simulink model "ofdm_txrx_mimo_2x"  (Simulink 6.6)
settings: Solver=VariableStepDiscrete  StartTime=0.0  StopTime=20e3  RelTol=1e-3
PreLoadFcn:
  | ofdm_rx_mimo_init
InitFcn:
  | ofdm_rx_mimo_init
StartFcn:
  | ofdm_rx_mimo_init
values from neighbouring files (symbols this model uses):
  BER_RAM_init_size = 2048    [ofdm_tx_mimo_init.m]
  CPLength = 16    [ofdm_tx_mimo_init.m]
  CRCPolynomial = hex2dec('04c11db7')    [ofdm_tx_mimo_init.m]
  freqOffset_track_filtCoef_i = hex2dec('2500')/2^32    [ofdm_rx_mimo_init.m]
  freqOffset_track_filtCoef_p = hex2dec('d0000')/2^32    [ofdm_rx_mimo_init.m]
  hi = zeros(1,length(cci))    [ofdm_rx_mimo_init.m]
  hr = zeros(1,length(ccr))    [ofdm_rx_mimo_init.m]
  max_CP_length = 16    [ofdm_tx_mimo_init.m]
  max_num_baseRateSymbols = 31    [ofdm_tx_mimo_init.m]
  max_num_subcarriers = 64    [ofdm_tx_mimo_init.m]
  max_num_trainingSymbols = 15    [ofdm_tx_mimo_init.m]
  max_numBytes = 16384    [ofdm_tx_mimo_init.m]
  max_OFDM_symbols = 2047    [ofdm_tx_mimo_init.m]
  modConstellation_qam16 = .75*[1 3 -1 -3]./3    [ofdm_tx_mimo_init.m]
  modConstellation_qam256 = 0.9375*[3 1 5 7 11 9 13 15 -3 -1 -5 -7 -11 -9 -13 -15]./15    [ofdm_tx_mimo_init.m]
  modConstellation_qam64 = 0.875*[3 1 5 7 -3 -1 -5 -7]./7    [ofdm_tx_mimo_init.m]
  modConstellation_qpsk = [1 -1]./sqrt(2)    [ofdm_tx_mimo_init.m]
  numBaseRateSymbols = 2    [ofdm_tx_mimo_init.m]
  numSubcarriers = 64    [ofdm_tx_mimo_init.m]
  numTrainingSymbols = 2    [ofdm_tx_mimo_init.m]
  pilot1_indicies = 7 + ( (64-7) * 2^16)    (= 3735559)    [ofdm_tx_mimo_init.m]
  pilot2_indicies = 21 + ( (64-21) * 2^16)    (= 2818069)    [ofdm_tx_mimo_init.m]
  pilotValue_neg = hex2dec('8000') + (2^16 * 0)    [ofdm_tx_mimo_init.m]
  pilotValue_pos = hex2dec('7FFF') + (2^16 * 0)    [ofdm_tx_mimo_init.m]
  pkt_crossCorr_thresh = 0.7    [ofdm_rx_mimo_init.m]
  pkt_energy_thresh = 0    [ofdm_rx_mimo_init.m]
  pkt_numPayloadBytes = 1400+42-6    (= 1436)    [ofdm_tx_mimo_init.m]
  pktByteNums = numHeaderBytes +  				byteIndex_numPayloadBytes(1)*2^8 +  				byteIndex_numPayloadBytes(2)*2^16 +  				byteIndex_simpleDynModMasks * 2^24    [ofdm_rx_mimo_init.m]
  PktDet_Delay = 26 + Symbol_Timing_Offset*2^7    (= 1050)    [ofdm_rx_mimo_init.m]
  preamble = 6*[0 shortsyms_10 longsyms_2]    [ofdm_tx_mimo_init.m]
  preamble_I = real(preamble)    [ofdm_tx_mimo_init.m]
  preamble_Q = imag(preamble)    [ofdm_tx_mimo_init.m]
  RAM_init_size = max_numBytes    (= 16384)    [ofdm_tx_mimo_init.m]
  reg_Interrupt_PktBuf_Control = reg_InterruptControl +  	1 * 2^16 +  	0 * 2^24    (= 65656)    [ofdm_rx_mimo_init.m]
  rx_controlBits = 1 * 2  		 + 1 * 4  		 + 0 * 8  		 + 1 * 16 * rx_SISO_Mode  		 + 1 * 32  		 + 1 * 64  		 + 0 * 128  		 + 1 * 256  		 + 0 * 512  		 + 1 * 1024  		 + 0 * 2048  		 + 1 * 4096  		 + 1 * 8192  		 + 0 * 16384  		 + 1 * 65536  		 + 1 * 131072  		 + 0 * 262144  		 + 1 * 524288    [ofdm_rx_mimo_init.m]
  Rx_PhaseNoiseTrack_K = 0.6094    [ofdm_rx_mimo_init.m]
  rx_postEq_scaling = round(rxScaling*2^11) * (1 + 2^16)    [ofdm_rx_mimo_init.m]
  RxDataScrambling_Seq = [40 198 78 63 82 173 102 245 48 111 172 115 147 230 216 93 72 65 62 2 205 242 122 90 128 83 105 97 73 10 5 252]    [ofdm_tx_mimo_init.m]
  simOnly_numSamples = length(preamble)+( (numSubcarriers+CPLength)*(numTrainingSymbols + numBaseRateSymbols + numFullRateSymbols/2) )    [ofdm_tx_mimo_init.m]
  train = [train train]    [ofdm_tx_mimo_init.m]
  tx_controlBits = (antB_preambleShift * 2^4) + (2^0 * tx_SISO_Mode) + 4*0 + 8*1    (= 73)    [ofdm_tx_mimo_init.m]
  TxDataScrambling_Seq = [40 198 78 63 82 173 102 245 48 111 172 115 147 230 216 93 72 65 62 2 205 242 122 90 128 83 105 97 73 10 5 252]    [ofdm_tx_mimo_init.m]
  txReg_symbolCounts = (2^16 * numFullRateSymbols) + (2^8 *numBaseRateSymbols ) + numTrainingSymbols    [ofdm_tx_mimo_init.m]
  TxRx_FFTScaling = bin2dec('010111') + (bin2dec('000101') * 2^6)    [ofdm_tx_mimo_init.m]
undefined symbols (not defined in the file or in its folder): AntA, AntB, BaseRate, CRC_table_gen, gcbh, intfilt, pktTiming_count_payloadStart, pktTiming_symbol_length, xlBlockLoadCallback, xlGetSimulinkPeriod
libraries: xbsIndex_r4 x3973, dspsigops x4, commchan3 x2, commrflib2 x2, dspsrcs4 x1
(part 13 of 38)

== system ofdm_txrx_mimo_2x/OFDM Tx MIMO/OutputBuffers/Addr Gen ==
  TrainingSym : Inport
  BaseRateSym : Inport  Port=2
  SymWrDone : Inport  Port=3
  Xk Index : Inport  Port=4
  rst : Inport  Port=5
  1 Shift Reg : Subsystem
  1 Shift Reg1 : Subsystem
  2 Shift Reg : Subsystem
  6LSB1 : xbsIndex_r4/Slice  nbits=log2(numSubcarriers)  boolean_output=off  mode=Lower Bit Location + Width  bit1=0  base1=MSB of Input  bit0=0  base0=LSB of Input  dbl_ovrd=off  block_type=slice
      settings: infoedit="Extracts a given range of bits from each input sample and presents it at the output.  The output type is ordinarily unsigned with binary point at zero, but can be Boolean when the slice is one bit wide.<P><P>Hardware notes: In hardware this block costs nothing."  block_version=VER_STRING_GOES_HERE
  AddSub : xbsIndex_r4/AddSub  mode=Addition  use_carryin=off  use_carryout=off  en=off  latency=0  precision=Full  arith_type=Unsigned  n_bits=16  bin_pt=14  quantization=Truncate  overflow=Wrap  dbl_ovrd=off  use_behavioral_HDL=off  pipelined=off  use_rpm=on  hw_selection=Fabric  block_type=addsub
      settings: block_version=VER_STRING_GOES_HERE
  Addr Gen Scope : Scope  NumInputPorts=6
      settings: TimeRange=6000  YMin=0~0~-0.1~-0.1~-0.1~-0.1  YMax=150~150~1.1~1.1~1.1~1.1  SaveName=ScopeData6
  Addr Gen Scope1 : Scope  NumInputPorts=6
      settings: TimeRange=6000  YMin=0~0~-0.1~-0.1~-0.1~-0.1  YMax=150~150~1.1~1.1~1.1~1.1  SaveName=ScopeData37
  Concat : xbsIndex_r4/Concat  num_inputs=2  dbl_ovrd=off  block_type=concat
      settings: block_version=VER_STRING_GOES_HERE
  Concat1 : xbsIndex_r4/Concat  num_inputs=2  dbl_ovrd=off  block_type=concat
      settings: block_version=VER_STRING_GOES_HERE
  Constant1 : xbsIndex_r4/Constant  arith_type=Boolean  const=1  n_bits=1  bin_pt=0  explicit_period=off  period=2  equ=P=C  opselect=C  inp2=PCIN>>17  opr=+  inp1=P  carry=CIN  dbl_ovrd=off  block_type=constant
      settings: block_version=VER_STRING_GOES_HERE
  Convert : xbsIndex_r4/Convert  arith_type=Boolean  n_bits=16  bin_pt=14  quantization=Truncate  overflow=Wrap  latency=0  dbl_ovrd=off  pipeline=off  block_type=convert
      settings: block_version=VER_STRING_GOES_HERE
  Delay : xbsIndex_r4/Delay  en=off  latency=1  dbl_ovrd=off  reg_retiming=off  block_type=delay
      settings: infoedit=Hardware notes: A delay line is a chain, each link of which is an SRL16 followed by a flip-flop. If register retiming is enabled, the delay line is a chain of flip-flops.  block_version=VER_STRING_GOES_HERE
  Down Sample : xbsIndex_r4/Down Sample  sample_ratio=2  sample_phase=First Value of Frame  en=off  latency=0  dbl_ovrd=off  block_type=dsamp
      settings: block_version=VER_STRING_GOES_HERE
  Down Sample1 : xbsIndex_r4/Down Sample  sample_ratio=2  sample_phase=First Value of Frame  en=off  latency=1  dbl_ovrd=off  block_type=dsamp
      settings: block_version=VER_STRING_GOES_HERE
  Down Sample2 : xbsIndex_r4/Down Sample  sample_ratio=2  sample_phase="Last Value of Frame  (most efficient)"  en=off  latency=1  dbl_ovrd=off  block_type=dsamp
      settings: block_version=VER_STRING_GOES_HERE
  Inverter : xbsIndex_r4/Inverter  en=off  latency=0  dbl_ovrd=off  block_type=inv
      settings: block_version=VER_STRING_GOES_HERE
  Inverter1 : xbsIndex_r4/Inverter  en=off  latency=0  dbl_ovrd=off  block_type=inv
      settings: block_version=VER_STRING_GOES_HERE
  Inverter2 : xbsIndex_r4/Inverter  en=off  latency=0  dbl_ovrd=off  block_type=inv
      settings: block_version=VER_STRING_GOES_HERE
  Logical : xbsIndex_r4/Logical  logical_function=AND  inputs=2  en=off  latency=0  precision=Full  arith_type=Unsigned  n_bits=16  bin_pt=0  align_bp=on  dbl_ovrd=off  block_type=logical
      settings: block_version=VER_STRING_GOES_HERE
  Logical1 : xbsIndex_r4/Logical  logical_function=AND  inputs=2  en=off  latency=0  precision=Full  arith_type=Unsigned  n_bits=16  bin_pt=0  align_bp=on  dbl_ovrd=off  block_type=logical
      settings: block_version=VER_STRING_GOES_HERE
  Logical2 : xbsIndex_r4/Logical  logical_function=OR  inputs=2  en=off  latency=0  precision=Full  arith_type=Unsigned  n_bits=16  bin_pt=0  align_bp=on  dbl_ovrd=off  block_type=logical
      settings: block_version=VER_STRING_GOES_HERE
  Logical3 : xbsIndex_r4/Logical  logical_function=AND  inputs=2  en=off  latency=0  precision=Full  arith_type=Unsigned  n_bits=16  bin_pt=0  align_bp=on  dbl_ovrd=off  block_type=logical
      settings: block_version=VER_STRING_GOES_HERE
  Oscillator : Subsystem
  Oscillator1 : Subsystem
  Rd Counter : Subsystem
  SR Latch : Subsystem
  Up Sample : xbsIndex_r4/Up Sample  sample_ratio=2  copy_samples=off  dbl_ovrd=off  block_type=usamp
      settings: infoedit="Up samples input data.  Inserted values  can be zeros or copies of the most recent input sample.<P><P>Hardware notes: No hardware is needed if inserted values are copies of the input sample; otherwise, a mux and single bit flip-flop are used."  block_version=VER_STRING_GOES_HERE
  LastSamp : Outport
  OE#A : Outport  Port=2
  OE#B : Outport  Port=3
  Wr Addr : Outport  Port=4
  Rd Addr : Outport  Port=5
  Rd Vout : Outport  Port=6
  wires:
  Down Sample/1 -> Concat1/1, Addr Gen Scope/4  "Rd Bank Sel"
  Rd Counter/1(Q) -> Concat1/2
  Constant1/1 -> 2 Shift Reg/1(d)
  2 Shift Reg/1(Q) -> Rd Counter/1(En), SR Latch/1(S)
  Down Sample2/1 -> Rd Counter/2(Rst), 2 Shift Reg/3(rst), SR Latch/2(R)
  AddSub/1 -> 6LSB1/1
  6LSB1/1 -> Concat/2
  [regRX_CP_Length] -> AddSub/2
  Down Sample1/1 -> 2 Shift Reg/2(en)
  rst/1 -> Oscillator/2(rst), 1 Shift Reg/2(rst), Down Sample2/1, Oscillator1/2(rst), 1 Shift Reg1/2(rst), Addr Gen Scope1/1
  Oscillator/1(Q) -> Inverter/1, Concat/1, Addr Gen Scope/3  "Wr Bank Sel"
  SymWrDone/1 -> Oscillator/3(en), Down Sample1/1, Addr Gen Scope1/2
  Concat/1 -> Wr Addr/1, Addr Gen Scope/1  "Write Addr"
  Xk Index/1 -> AddSub/1, Addr Gen Scope1/4
  Concat1/1 -> Rd Addr/1, Addr Gen Scope/2  "Read Addr"
  SR Latch/1(Q) -> Rd Vout/1
  1 Shift Reg/1(Q) -> Down Sample/1
  Rd Counter/2(Ovfl) -> Up Sample/1
  Up Sample/1 -> Delay/1
  Delay/1 -> 1 Shift Reg/3(en), Oscillator1/1(en), 1 Shift Reg1/3(en), LastSamp/1
  Inverter/1 -> 1 Shift Reg/1(d)
  BaseRateSym/1 -> Oscillator/1(period), Addr Gen Scope1/3
  Oscillator1/1(Q) -> Inverter1/1, Logical/2
  Inverter1/1 -> Logical1/1
  [TxReg_SISO_Mode] -> Convert/1
  Logical/1 -> Addr Gen Scope/5, Logical3/1  "Ant A Disable"
  Logical1/1 -> Logical2/2, Addr Gen Scope/6  "Ant B Disable"
  TrainingSym/1 -> 1 Shift Reg1/1(d)
  1 Shift Reg1/1(Q) -> Logical/1, Logical1/2
  Logical2/1 -> OE#B/1
  Convert/1 -> Inverter2/1, Logical2/1
  Logical3/1 -> OE#A/1
  Inverter2/1 -> Logical3/2

== system ofdm_txrx_mimo_2x/OFDM Tx MIMO/OutputBuffers/Mem Control ==
  note: The IFFT of the training symbols is only computed once per antenna, regardless
      | of the number of actual copies of training symbols that are transmitted. This is why
      | a constant 2 is used to decide whether the current IFFT core output symbol
      | is a training symbol or not- the third IFFT output is guaranteed to be a base-rate symbol.
  FFTsymNum : Inport
  1LSB : xbsIndex_r4/Slice  nbits=1  boolean_output=off  mode=Lower Bit Location + Width  bit1=0  base1=MSB of Input  bit0=0  base0=LSB of Input  dbl_ovrd=off  block_type=slice
      settings: infoedit="Extracts a given range of bits from each input sample and presents it at the output.  The output type is ordinarily unsigned with binary point at zero, but can be Boolean when the slice is one bit wide.<P><P>Hardware notes: In hardware this block costs nothing."  block_version=VER_STRING_GOES_HERE
  AddSub1 : xbsIndex_r4/AddSub  mode=Addition  use_carryin=off  use_carryout=off  en=off  latency=0  precision=Full  arith_type=Unsigned  n_bits=16  bin_pt=14  quantization=Truncate  overflow=Wrap  dbl_ovrd=off  use_behavioral_HDL=off  pipelined=off  use_rpm=on  hw_selection=Fabric  block_type=addsub
      settings: block_version=VER_STRING_GOES_HERE
  AddSub2 : xbsIndex_r4/AddSub  mode=Addition  use_carryin=off  use_carryout=off  en=off  latency=0  precision=Full  arith_type=Unsigned  n_bits=16  bin_pt=14  quantization=Truncate  overflow=Wrap  dbl_ovrd=off  use_behavioral_HDL=off  pipelined=off  use_rpm=on  hw_selection=Fabric  block_type=addsub
      settings: block_version=VER_STRING_GOES_HERE
  Constant1 : xbsIndex_r4/Constant  arith_type=Unsigned  const=2  n_bits=2  bin_pt=0  explicit_period=off  period=1  equ=P=C  opselect=C  inp2=PCIN>>17  opr=+  inp1=P  carry=CIN  dbl_ovrd=off  block_type=constant
      settings: block_version=VER_STRING_GOES_HERE
  Constant2 : xbsIndex_r4/Constant  arith_type=Unsigned  const=2  n_bits=2  bin_pt=0  explicit_period=off  period=1  equ=P=C  opselect=C  inp2=PCIN>>17  opr=+  inp1=P  carry=CIN  dbl_ovrd=off  block_type=constant
      settings: block_version=VER_STRING_GOES_HERE
  Constant3 : xbsIndex_r4/Constant  arith_type=Unsigned  const=2  n_bits=2  bin_pt=0  explicit_period=off  period=1  equ=P=C  opselect=C  inp2=PCIN>>17  opr=+  inp1=P  carry=CIN  dbl_ovrd=off  block_type=constant
      settings: block_version=VER_STRING_GOES_HERE
  Convert : xbsIndex_r4/Convert  arith_type=Boolean  n_bits=16  bin_pt=14  quantization=Truncate  overflow=Wrap  latency=0  dbl_ovrd=off  pipeline=off  block_type=convert
      settings: block_version=VER_STRING_GOES_HERE
  Inverter : xbsIndex_r4/Inverter  en=off  latency=0  dbl_ovrd=off  block_type=inv
      settings: block_version=VER_STRING_GOES_HERE
  Logical1 : xbsIndex_r4/Logical  logical_function=AND  inputs=2  en=off  latency=0  precision=Full  arith_type=Unsigned  n_bits=16  bin_pt=0  align_bp=on  dbl_ovrd=off  block_type=logical
      settings: block_version=VER_STRING_GOES_HERE
  Logical2 : xbsIndex_r4/Logical  logical_function=OR  inputs=2  en=off  latency=0  precision=Full  arith_type=Unsigned  n_bits=16  bin_pt=0  align_bp=on  dbl_ovrd=off  block_type=logical
      settings: block_version=VER_STRING_GOES_HERE
  Logical3 : xbsIndex_r4/Logical  logical_function=OR  inputs=2  en=off  latency=0  precision=Full  arith_type=Unsigned  n_bits=16  bin_pt=0  align_bp=on  dbl_ovrd=off  block_type=logical
      settings: block_version=VER_STRING_GOES_HERE
  Output Scope1 : Scope  NumInputPorts=6
      settings: TimeRange=2250  YMin=0~0~0~0~0~-5  YMax=1~1~1~1~14~5  SaveName=ScopeData9
  Relational1 : xbsIndex_r4/Relational  mode=a>=b  en=off  latency=0  dbl_ovrd=off  block_type=relational
      settings: xl_area=[2 0 0 4 0 0 0]  block_version=VER_STRING_GOES_HERE
  Relational2 : xbsIndex_r4/Relational  mode=a<=b  en=off  latency=0  dbl_ovrd=off  block_type=relational
      settings: xl_area=[2 0 0 4 0 0 0]  block_version=VER_STRING_GOES_HERE
  Relational3 : xbsIndex_r4/Relational  mode=a=b  en=off  latency=0  dbl_ovrd=off  block_type=relational
      settings: xl_area=[2 0 0 4 0 0 0]  block_version=VER_STRING_GOES_HERE
  Relational4 : xbsIndex_r4/Relational  mode=a<b  en=off  latency=0  dbl_ovrd=off  block_type=relational
      settings: xl_area=[2 0 0 4 0 0 0]  block_version=VER_STRING_GOES_HERE
  Shift : xbsIndex_r4/Shift  shift_dir=Right  shift_bits=1  en=off  latency=0  precision=Full  arith_type="Signed  (2's comp)"  n_bits=16  bin_pt=14  quantization=Truncate  overflow=Wrap  dbl_ovrd=off  block_type=shift
      settings: block_version=VER_STRING_GOES_HERE
  LastSym : Outport
  weA : Outport  Port=2
  weB : Outport  Port=3
  TrainingSym : Outport  Port=4
  BaseRateSym : Outport  Port=5
  wires:
  Constant1/1 -> Relational1/2
  [reg_numBaseRateSyms] -> Shift/1
  AddSub1/1 -> Relational4/2, AddSub2/1
  Constant3/1 -> AddSub1/2
  FFTsymNum/1 -> 1LSB/1, Relational4/1, Relational1/1, Relational2/1, Output Scope1/5, Relational3/1
  Relational4/1 -> Logical1/1
  Relational1/1 -> Logical1/2
  1LSB/1 -> Convert/1
  Convert/1 -> Inverter/1, Logical3/1
  Inverter/1 -> Logical2/2
  Logical2/1 -> weA/1, Output Scope1/1
  Logical3/1 -> weB/1, Output Scope1/2
  Logical1/1 -> Logical3/2, Logical2/1, BaseRateSym/1, Output Scope1/3
  Shift/1 -> AddSub1/1
  Constant2/1 -> Relational2/2
  Relational2/1 -> TrainingSym/1, Output Scope1/4
  [reg_numFullRateSyms] -> AddSub2/2
  AddSub2/1 -> Relational3/2
  Relational3/1 -> LastSym/1, Output Scope1/6

== system ofdm_txrx_mimo_2x/OFDM Tx MIMO/OutputBuffers/RAMs ==
  A We : Inport
  B We : Inport  Port=2
  A OE# : Inport  Port=3
  B OE# : Inport  Port=4
  Wr Addr : Inport  Port=5
  Rd Addr : Inport  Port=6
  Rd Vin : Inport  Port=7
  I : Inport  Port=8
  Q : Inport  Port=9
  Antenna A RAM : Subsystem
  Antenna B RAM : Subsystem
  Down Sample{,1..3} (4 alike) : xbsIndex_r4/Down Sample  sample_ratio=2  sample_phase="Last Value of Frame  (most efficient)"  en=off  latency=1  dbl_ovrd=off  block_type=dsamp
      settings: block_version=VER_STRING_GOES_HERE
  Output Buffers Outputs : Scope  NumInputPorts=7
      settings: TimeRange=3000  YMin=-0.1~-0.15~-0.15~-0.125~0~0.95~-1  YMax=0.125~0.075~0.2~0.125~150~1.05~1  SaveName=ScopeData31
  done{1..7} (7 alike) : xbsIndex_r4/Gateway Out  hdl_port=off  timing_constraint=None  locs_specified=off  LOCs={}  block_type=gatewayout
      settings: infoedit="Gateway out block.  Converts Xilinx fixed point inputs into ouputs of type Simulink integer, double, or fixed point.<P><P>Hardware notes:  In hardware these blocks become top level output ports or are discarded, depending on how they are configured."  xl_area=[0 0 0 0 1 0 0]  block_version=VER_STRING_GOES_HERE
  AntA_I : Outport
  AntA_Q : Outport  Port=2
  AntB_I : Outport  Port=3
  AntB_Q : Outport  Port=4
  Rd Vout : Outport  Port=5
  wires:
  Antenna B RAM/2(" Q") -> Down Sample/1
  Antenna B RAM/1(" I") -> Down Sample1/1
  Rd Addr/1 -> Antenna A RAM/5(Rd Addr), done2/1, Antenna B RAM/5(Rd Addr)
  Antenna A RAM/2(" Q") -> Down Sample2/1
  Antenna A RAM/1(" I") -> Down Sample3/1
  Wr Addr/1 -> Antenna A RAM/4(Wr Addr), Antenna B RAM/4(Wr Addr)
  I/1 -> Antenna A RAM/1(I), Antenna B RAM/1(I)
  Q/1 -> Antenna A RAM/2(Q), Antenna B RAM/2(Q)
  A We/1 -> Antenna A RAM/3(We)
  B We/1 -> Antenna B RAM/3(We)
  B OE#/1 -> Antenna B RAM/6(OE#)
  A OE#/1 -> Antenna A RAM/6(OE#), done7/1
  Down Sample/1 -> AntB_Q/1, done3/1
  Down Sample1/1 -> AntB_I/1, done4/1
  Down Sample2/1 -> AntA_Q/1, done5/1
  Down Sample3/1 -> AntA_I/1, done6/1
  Rd Vin/1 -> done1/1, Rd Vout/1
  done7/1 -> Output Buffers Outputs/7
  done1/1 -> Output Buffers Outputs/6
  done2/1 -> Output Buffers Outputs/5
  done3/1 -> Output Buffers Outputs/4
  done4/1 -> Output Buffers Outputs/3
  done5/1 -> Output Buffers Outputs/2
  done6/1 -> Output Buffers Outputs/1

== system ofdm_txrx_mimo_2x/OFDM Tx MIMO/OutputBuffers/negedge ==
  D : Inport
  Delay : xbsIndex_r4/Delay  en=off  latency=1  dbl_ovrd=off  reg_retiming=on  block_type=delay
      settings: infoedit=Hardware notes: A delay line is a chain, each link of which is an SRL16 followed by a flip-flop. If register retiming is enabled, the delay line is a chain of flip-flops.  block_version=VER_STRING_GOES_HERE
  Inverter1 : xbsIndex_r4/Inverter  en=off  latency=0  dbl_ovrd=off  block_type=inv
      settings: block_version=VER_STRING_GOES_HERE
  Logical3 : xbsIndex_r4/Logical  logical_function=AND  inputs=2  en=off  latency=0  precision=Full  arith_type=Unsigned  n_bits=16  bin_pt=0  align_bp=on  dbl_ovrd=off  block_type=logical
      settings: block_version=VER_STRING_GOES_HERE
  Q : Outport
  wires:
  Logical3/1 -> Q/1
  Delay/1 -> Logical3/2
  D/1 -> Delay/1, Inverter1/1
  Inverter1/1 -> Logical3/1

== system ofdm_txrx_mimo_2x/OFDM Tx MIMO/OutputMuxes/Preamble Generation ==
  Addr : Inport
  2-bit Counter : xbsIndex_r4/Counter  cnt_type=Free Running  cnt_to=Inf  operation=Up  start_count=0  cnt_by_val=1  arith_type=Unsigned  n_bits=2  bin_pt=0  load_pin=off  rst=off  en=off  explicit_period=on  period=1  dbl_ovrd=off  use_behavioral_HDL=off  use_rpm=off  implementation=Fabric  block_type=counter
      settings: block_version=VER_STRING_GOES_HERE
  2x : Subsystem
  2x1 : Subsystem
  2x2 : Subsystem
  2x3 : Subsystem
  A btwn B&C : Subsystem
  A btwn B&C1 : Subsystem
  Ant B Addr : Subsystem
  Constant : xbsIndex_r4/Constant  arith_type=Boolean  const=0  n_bits=1  bin_pt=0  explicit_period=off  period=1  equ=P=C  opselect=C  inp2=PCIN>>17  opr=+  inp1=P  carry=CIN  dbl_ovrd=off  block_type=constant
      settings: block_version=8.2.02
  Constant1 : xbsIndex_r4/Constant  arith_type=Signed (2's comp)  const=0  n_bits=14  bin_pt=13  explicit_period=off  period=1  equ=P=C  opselect=C  inp2=PCIN>>17  opr=+  inp1=P  carry=CIN  dbl_ovrd=off  block_type=constant
      settings: block_version=8.2.02
  Down Sample : xbsIndex_r4/Down Sample  sample_ratio=2  sample_phase="Last Value of Frame  (most efficient)"  en=off  latency=1  dbl_ovrd=off  block_type=dsamp
      settings: block_version=VER_STRING_GOES_HERE
  Logical1 : xbsIndex_r4/Logical  logical_function=OR  inputs=2  en=off  latency=0  precision=Full  arith_type=Unsigned  n_bits=8  bin_pt=2  align_bp=on  dbl_ovrd=off  block_type=logical
      settings: xl_area=[1 0 0 1 0 0 0]  block_version=VER_STRING_GOES_HERE
  Mult : xbsIndex_r4/Mult  precision=User Defined  arith_type="Signed  (2's comp)"  n_bits=14  bin_pt=13  quantization=Truncate  overflow=Wrap  en=off  latency=2  dbl_ovrd=off  use_behavioral_HDL=off  use_embedded=on  opt=Speed  optimum_pipeline=off  pipeline=on  use_rpm=on  placement_style=Rectangular shape  block_type=mult
      settings: infoedit=Hardware notes: To use the internal pipeline stage of the dedicated multiplier you must select 'Pipeline for maximum performance'.  block_version=VER_STRING_GOES_HERE
  Mux : xbsIndex_r4/Mux  inputs=4  en=off  latency=2  precision=Full  arith_type=Unsigned  n_bits=16  bin_pt=14  quantization=Truncate  overflow=Wrap  dbl_ovrd=off  block_type=mux
      settings: block_version=VER_STRING_GOES_HERE
  Preamble_I : xbsIndex_r4/Dual Port RAM  depth=length(preamble_I)  initVector=preamble_I  distributed_mem=Block RAM  init_a=0  init_b=0  rst_a=on  rst_b=on  en_a=off  en_b=off  latency=1  write_mode_A=Read After Write  write_mode_B=Read After Write  dbl_ovrd=off  optimize=Area  use_rpm=on  block_type=dpram
      settings: block_version=8.2.01
  Preamble_Q : xbsIndex_r4/Dual Port RAM  depth=length(preamble_Q)  initVector=preamble_Q  distributed_mem=Block RAM  init_a=0  init_b=0  rst_a=on  rst_b=on  en_a=off  en_b=off  latency=1  write_mode_A=Read After Write  write_mode_B=Read After Write  dbl_ovrd=off  optimize=Area  use_rpm=on  block_type=dpram
      settings: block_version=8.2.01
  Slice1 : xbsIndex_r4/Slice  nbits=8  boolean_output=off  mode=Lower Bit Location + Width  bit1=0  base1=MSB of Input  bit0=0  base0=LSB of Input  dbl_ovrd=off  block_type=slice
      settings: infoedit="Extracts a given range of bits from each input sample and presents it at the output.  The output type is ordinarily unsigned with binary point at zero, but can be Boolean when the slice is one bit wide.<P><P>Hardware notes: In hardware this block costs nothing."  block_version=VER_STRING_GOES_HERE
  Slice2 : xbsIndex_r4/Slice  nbits=8  boolean_output=off  mode=Lower Bit Location + Width  bit1=0  base1=MSB of Input  bit0=8  base0=LSB of Input  dbl_ovrd=off  block_type=slice
      settings: infoedit="Extracts a given range of bits from each input sample and presents it at the output.  The output type is ordinarily unsigned with binary point at zero, but can be Boolean when the slice is one bit wide.<P><P>Hardware notes: In hardware this block costs nothing."  block_version=VER_STRING_GOES_HERE
  Slice3 : xbsIndex_r4/Slice  nbits=8  boolean_output=off  mode=Lower Bit Location + Width  bit1=0  base1=MSB of Input  bit0=16  base0=LSB of Input  dbl_ovrd=off  block_type=slice
      settings: infoedit="Extracts a given range of bits from each input sample and presents it at the output.  The output type is ordinarily unsigned with binary point at zero, but can be Boolean when the slice is one bit wide.<P><P>Hardware notes: In hardware this block costs nothing."  block_version=VER_STRING_GOES_HERE
  Slice4 : xbsIndex_r4/Slice  nbits=8  boolean_output=off  mode=Lower Bit Location + Width  bit1=0  base1=MSB of Input  bit0=24  base0=LSB of Input  dbl_ovrd=off  block_type=slice
      settings: infoedit="Extracts a given range of bits from each input sample and presents it at the output.  The output type is ordinarily unsigned with binary point at zero, but can be Boolean when the slice is one bit wide.<P><P>Hardware notes: In hardware this block costs nothing."  block_version=VER_STRING_GOES_HERE
  Time Division Demultiplexer : xbsIndex_r4/Time Division Demultiplexer  frame_pattern=[1 1 1 1]  impl_style=Multiple Channel  vin=off  dbl_ovrd=off  explicit_period=off  period=1  block_type=tdd
      settings: infoedit=Samples presented at the input are selected and down-sampled according to the frame sampling pattern specified. The sampled input is presented either as a single or multiple channel at the output.  block_version=VER_STRING_GOES_HERE
  Up Sample{,1..3} (4 alike) : xbsIndex_r4/Up Sample  sample_ratio=4  copy_samples=on  dbl_ovrd=off  block_type=usamp
      settings: infoedit="Up samples input data.  Inserted values  can be zeros or copies of the most recent input sample.<P><P>Hardware notes: No hardware is needed if inserted values are copies of the input sample; otherwise, a mux and single bit flip-flop are used."  block_version=VER_STRING_GOES_HERE
  AntA_I : Outport
  AntA_Q : Outport  Port=2
  AntB_I : Outport  Port=3
  AntB_Q : Outport  Port=4
  wires:
  Addr/1 -> A btwn B&C/1(A), A btwn B&C1/1(A), Ant B Addr/1(A-Addr), Preamble_I/1, Preamble_Q/1
  2-bit Counter/1 -> Mux/1
  Mux/1 -> Mult/2
  [TxReg_PreambleScaling] -> Mult/1
  Up Sample/1 -> Mux/2
  Up Sample1/1 -> Mux/3
  Up Sample2/1 -> Mux/4
  Up Sample3/1 -> Mux/5
  Time Division Demultiplexer/1 -> AntA_I/1
  Time Division Demultiplexer/2 -> AntA_Q/1
  Time Division Demultiplexer/3 -> AntB_I/1
  Time Division Demultiplexer/4 -> AntB_Q/1
  Mult/1 -> Time Division Demultiplexer/1
  [TxReg_PreambleMasking] -> Slice1/1, Slice2/1, Slice3/1, Slice4/1
  Slice1/1 -> 2x/1(I)
  Slice3/1 -> 2x2/1(I)
  Slice4/1 -> 2x3/1(I)
  Slice2/1 -> 2x1/1(I)
  2x/1(O) -> [AntA_Start]
  2x1/1(O) -> [AntA_Stop]
  2x2/1(O) -> [AntB_Start]
  2x3/1(O) -> [AntB_Stop]
  [AntA_Start] -> A btwn B&C/2(B)
  [AntA_Stop] -> A btwn B&C/3(C)
  A btwn B&C1/1(Q) -> Logical1/1
  [AntB_Start] -> A btwn B&C1/2(B)
  [AntB_Stop] -> A btwn B&C1/3(C)
  Constant1/1 -> Preamble_I/2, Preamble_I/5, Preamble_Q/2, Preamble_Q/5
  Logical1/1 -> Down Sample/1
  [TxReg_DisableAntBPreamble] -> Logical1/2
  Down Sample/1 -> Preamble_Q/8, Preamble_I/8
  A btwn B&C/1(Q) -> Preamble_I/7, Preamble_Q/7
  Ant B Addr/1(B-Addr) -> Preamble_Q/4, Preamble_I/4
  Preamble_I/1 -> Up Sample/1
  Preamble_Q/1 -> Up Sample1/1
  Preamble_I/2 -> Up Sample2/1
  Preamble_Q/2 -> Up Sample3/1
  Constant/1 -> Preamble_I/6, Preamble_I/3, Preamble_Q/6, Preamble_Q/3

== system ofdm_txrx_mimo_2x/OFDM Tx MIMO/Training_Data/Ant Selector ==
  note: Antenna selction should switch with
      | alternate OFDM symbols during full-rate
      | paylod symbols. During training
      | and base rate symbols there is no
      | need to switch.
  BaseRate : Inport
  CP#_Data : Inport  Port=2
  Inverter2 : xbsIndex_r4/Inverter  en=off  latency=0  dbl_ovrd=off  block_type=inv
      settings: block_version=VER_STRING_GOES_HERE
  Logical1 : xbsIndex_r4/Logical  logical_function=AND  inputs=2  en=off  latency=0  precision=Full  arith_type=Unsigned  n_bits=16  bin_pt=0  align_bp=on  dbl_ovrd=off  block_type=logical
      settings: block_version=VER_STRING_GOES_HERE
  Oscillator : Subsystem
  negedge : Subsystem
  Sel : Outport
  wires:
  BaseRate/1 -> Inverter2/1, Oscillator/1(rst)
  CP#_Data/1 -> negedge/1(D)
  Oscillator/1(Q) -> Sel/1
  negedge/1(Q) -> Logical1/2
  Logical1/1 -> Oscillator/2(en)
  Inverter2/1 -> Logical1/1

== system ofdm_txrx_mimo_2x/OFDM Tx MIMO/Training_Data/FlexibleMod ==
  ifft_index : Inport
  packet_done : Inport  Port=2
  vin : Inport  Port=3
  start : Inport  Port=4
  BaseRate_En : Inport  Port=5
  ant_sel : Inport  Port=6
  DataModulation : Subsystem
  Logical1 : xbsIndex_r4/Logical  logical_function=OR  inputs=2  en=off  latency=0  precision=Full  arith_type=Unsigned  n_bits=8  bin_pt=2  align_bp=on  dbl_ovrd=off  block_type=logical
      settings: xl_area=[1 0 0 1 0 0 0]  block_version=VER_STRING_GOES_HERE
  S-R Latch : Subsystem
  posedge : Subsystem  mask{period=1}
  I : Outport
  Q : Outport  Port=2
  wires:
  DataModulation/2(I) -> I/1
  DataModulation/1(Q) -> Q/1
  posedge/1(pulse) -> DataModulation/1(ready)
  S-R Latch/1(Q) -> posedge/1(signal)
  Logical1/1 -> S-R Latch/1(R)
  ifft_index/1 -> DataModulation/2(ifft_index)
  vin/1 -> DataModulation/3(valid)
  start/1 -> S-R Latch/2(S)
  [TxReset] -> Logical1/2
  packet_done/1 -> Logical1/1
  BaseRate_En/1 -> DataModulation/4(BaseRate_En)
  ant_sel/1 -> DataModulation/5(ant_sel)

== system ofdm_txrx_mimo_2x/OFDM Tx MIMO/Training_Data/Training_Pilots ==
  note: The training seq lookup tables have latency=3 to
      | align their output with the IFFT's expected inputs.
      | The IFFT xn output runs three cycles ahead of the input
      | data (i.e. xn=3 when data[0] should be on the IFFT inputs.
  Xn_index : Inport
  I_mod : Inport  Port=2
  Q_mod : Inport  Port=3
  train# : Inport  Port=4
  antSel : Inport  Port=5
  rst : Inport  Port=6
  Concat : xbsIndex_r4/Concat  num_inputs=2  dbl_ovrd=off  block_type=concat
      settings: block_version=VER_STRING_GOES_HERE
  Convert : xbsIndex_r4/Convert  arith_type=Unsigned  n_bits=1  bin_pt=0  quantization=Truncate  overflow=Wrap  latency=0  dbl_ovrd=off  pipeline=off  block_type=convert
      settings: xl_area=[0, 0, 0, 0, 0, 0, 0]  block_version=VER_STRING_GOES_HERE
  Delay1 : xbsIndex_r4/Delay  en=off  latency=3  dbl_ovrd=off  reg_retiming=off  block_type=delay
      settings: infoedit=Hardware notes: A delay line is a chain, each link of which is an SRL16 followed by a flip-flop. If register retiming is enabled, the delay line is a chain of flip-flops.  xl_area=[1 1 0 0 0 0 0]  block_version=VER_STRING_GOES_HERE
  Inverter : xbsIndex_r4/Inverter  en=off  latency=0  dbl_ovrd=off  block_type=inv
      settings: xl_area=[0, 0, 0, 0, 0, 0, 0]  block_version=VER_STRING_GOES_HERE
  Mux : xbsIndex_r4/Mux  inputs=2  en=off  latency=0  precision=User Defined  arith_type="Signed  (2's comp)"  n_bits=16  bin_pt=15  quantization=Truncate  overflow=Wrap  dbl_ovrd=off  block_type=mux
      settings: xl_area=[0, 0, 0, 0, 0, 0, 0]  block_version=VER_STRING_GOES_HERE
  Mux1 : xbsIndex_r4/Mux  inputs=2  en=off  latency=0  precision=User Defined  arith_type="Signed  (2's comp)"  n_bits=16  bin_pt=15  quantization=Truncate  overflow=Wrap  dbl_ovrd=off  block_type=mux
      settings: xl_area=[0, 0, 0, 0, 0, 0, 0]  block_version=VER_STRING_GOES_HERE
  Scrambled_Pilot_Inserter : Subsystem
  Train_I : xbsIndex_r4/ROM  depth=2*numSubcarriers  initVector=real(train)  distributed_mem=Block RAM  rst=off  init_reg=0  en=on  latency=3  arith_type="Signed  (2's comp)"  n_bits=modConstellation_prec  bin_pt=modConstellation_bp  dbl_ovrd=off  optimize=Area  use_rpm=off  block_type=sprom
      settings: xl_area=[0, 0, 0, 0, 0, 0, 0]  block_version=VER_STRING_GOES_HERE
  Train_Q : xbsIndex_r4/ROM  depth=2*numSubcarriers  initVector=imag(train)  distributed_mem=Block RAM  rst=off  init_reg=0  en=on  latency=3  arith_type="Signed  (2's comp)"  n_bits=modConstellation_prec  bin_pt=modConstellation_bp  dbl_ovrd=off  optimize=Area  use_rpm=off  block_type=sprom
      settings: xl_area=[0, 0, 0, 0, 0, 0, 0]  block_version=VER_STRING_GOES_HERE
  tx_I : Outport
  tx_Q : Outport  Port=2
  wires:
  Convert/1 -> Delay1/1
  Mux1/1 -> tx_Q/1
  Mux/1 -> tx_I/1
  Scrambled_Pilot_Inserter/1(" I") -> Mux/3
  Scrambled_Pilot_Inserter/2(" Q") -> Mux1/3
  Train_I/1 -> Mux/2
  Train_Q/1 -> Mux1/2
  Inverter/1 -> Train_I/2, Train_Q/2
  Concat/1 -> Train_Q/1, Train_I/1
  Xn_index/1 -> Concat/2, Scrambled_Pilot_Inserter/1(IFFT Index)
  train#/1 -> Inverter/1, Convert/1
  antSel/1 -> Concat/1, Scrambled_Pilot_Inserter/5(Ant Sel)
  I_mod/1 -> Scrambled_Pilot_Inserter/2("I ")
  Q_mod/1 -> Scrambled_Pilot_Inserter/3("Q ")
  Delay1/1 -> Mux/1, Mux1/1
  rst/1 -> Scrambled_Pilot_Inserter/4(rst)

== system ofdm_txrx_mimo_2x/OFDM Tx MIMO/TxControl/2x ==
  I : Inport
  Concat1 : xbsIndex_r4/Concat  num_inputs=2  dbl_ovrd=off  block_type=concat
      settings: block_version=VER_STRING_GOES_HERE
  Constant : xbsIndex_r4/Constant  arith_type=Unsigned  const=0  n_bits=1  bin_pt=0  explicit_period=off  period=1  equ=P=C  opselect=C  inp2=PCIN>>17  opr=+  inp1=P  carry=CIN  dbl_ovrd=off  block_type=constant
      settings: block_version=VER_STRING_GOES_HERE
  O : Outport
  wires:
  Constant/1 -> Concat1/2
  I/1 -> Concat1/1
  Concat1/1 -> O/1

== system ofdm_txrx_mimo_2x/OFDM Tx MIMO/TxControl/2x1 ==
  I : Inport
  Concat1 : xbsIndex_r4/Concat  num_inputs=2  dbl_ovrd=off  block_type=concat
      settings: block_version=VER_STRING_GOES_HERE
  Constant : xbsIndex_r4/Constant  arith_type=Unsigned  const=0  n_bits=1  bin_pt=0  explicit_period=off  period=1  equ=P=C  opselect=C  inp2=PCIN>>17  opr=+  inp1=P  carry=CIN  dbl_ovrd=off  block_type=constant
      settings: block_version=VER_STRING_GOES_HERE
  O : Outport
  wires:
  Concat1/1 -> O/1
  I/1 -> Concat1/1
  Constant/1 -> Concat1/2

== system ofdm_txrx_mimo_2x/OFDM Tx MIMO/TxControl/FFT Start Gen ==
  note: First two symbols are always training and should be processed immediately, back-to-back. The same IFFT
      | output will be repeated numTrainingSyms times. After this, the IFFT processes the base-rate symbols. Because
      | each base-rate symbol is transmitted from both antennas, the IFFT waits a full output symbol period before processing
      | the next symbol. After the base rate symbols, the multiplexing of full-rate symbols begins, so the IFFT is triggered twice
      | per output symbol period (computing two output symbols- one per antenna- per period).
  SymNum : Inport
  AddSub1 : xbsIndex_r4/AddSub  mode=Addition  use_carryin=off  use_carryout=off  en=off  latency=0  precision=Full  arith_type=Unsigned  n_bits=10  bin_pt=0  quantization=Truncate  overflow=Wrap  dbl_ovrd=off  use_behavioral_HDL=on  pipelined=off  use_rpm=on  hw_selection=Fabric  block_type=addsub
      settings: xl_area=[0, 0, 0, 0, 0, 0, 0]  block_version=VER_STRING_GOES_HERE
  Constant9 : xbsIndex_r4/Constant  arith_type=Unsigned  const=1  n_bits=1  bin_pt=0  explicit_period=off  period=1  equ=P=C  opselect=C  inp2=PCIN>>17  opr=+  inp1=P  carry=CIN  dbl_ovrd=off  block_type=constant
      settings: block_version=VER_STRING_GOES_HERE
  Inverter : xbsIndex_r4/Inverter  en=off  latency=0  dbl_ovrd=off  block_type=inv
      settings: block_version=VER_STRING_GOES_HERE
  LSB : xbsIndex_r4/Slice  nbits=1  boolean_output=on  mode=Lower Bit Location + Width  bit1=0  base1=LSB of Input  bit0=0  base0=LSB of Input  dbl_ovrd=off  block_type=slice
      settings: infoedit="Extracts a given range of bits from each input sample and presents it at the output.  The output type is ordinarily unsigned with binary point at zero, but can be Boolean when the slice is one bit wide.<P><P>Hardware notes: In hardware this block costs nothing."  block_version=VER_STRING_GOES_HERE
  Logical : xbsIndex_r4/Logical  logical_function=OR  inputs=3  en=off  latency=0  precision=Full  arith_type=Unsigned  n_bits=16  bin_pt=0  align_bp=on  dbl_ovrd=off  block_type=logical
      settings: block_version=VER_STRING_GOES_HERE
  Logical1 : xbsIndex_r4/Logical  logical_function=AND  inputs=3  en=off  latency=0  precision=Full  arith_type=Unsigned  n_bits=16  bin_pt=0  align_bp=on  dbl_ovrd=off  block_type=logical
      settings: block_version=VER_STRING_GOES_HERE
  Output Scope2 : Scope  NumInputPorts=6
      settings: TimeRange=2200  YMin=0~0~0~-1~-1~-1  YMax=1~1~1~1~1~1  SaveName=ScopeData7
  Relational1 : xbsIndex_r4/Relational  mode=a>=b  en=off  latency=0  dbl_ovrd=off  block_type=relational
      settings: xl_area=[2 0 0 4 0 0 0]  block_version=VER_STRING_GOES_HERE
  Relational2 : xbsIndex_r4/Relational  mode=a<b  en=off  latency=0  dbl_ovrd=off  block_type=relational
      settings: xl_area=[2 0 0 4 0 0 0]  block_version=VER_STRING_GOES_HERE
  Relational3 : xbsIndex_r4/Relational  mode=a>=b  en=off  latency=0  dbl_ovrd=off  block_type=relational
      settings: xl_area=[2 0 0 4 0 0 0]  block_version=VER_STRING_GOES_HERE
  Relational9 : xbsIndex_r4/Relational  mode=a<=b  en=off  latency=0  dbl_ovrd=off  block_type=relational
      settings: xl_area=[2 0 0 4 0 0 0]  block_version=VER_STRING_GOES_HERE
  En : Outport
  wires:
  Constant9/1 -> Relational9/2
  SymNum/1 -> Relational9/1, Relational1/1, Relational2/1, LSB/1, Relational3/1, Output Scope2/1
  Relational9/1 -> Logical/1, Output Scope2/2
  Logical/1 -> En/1, Output Scope2/5
  [reg_numTrainingSyms] -> Relational1/2, AddSub1/1
  Relational1/1 -> Logical1/1
  AddSub1/1 -> Relational2/2, Relational3/2
  [reg_numBaseRateSyms] -> AddSub1/2
  Relational2/1 -> Logical1/2
  LSB/1 -> Inverter/1
  Logical1/1 -> Logical/2, Output Scope2/3
  Relational3/1 -> Logical/3, Output Scope2/4
  Inverter/1 -> Logical1/3

== system ofdm_txrx_mimo_2x/OFDM Tx MIMO/TxControl/Posedge1 ==
  D : Inport
  Delay : xbsIndex_r4/Delay  en=off  latency=1  dbl_ovrd=off  reg_retiming=off  block_type=delay
      settings: infoedit=Hardware notes: A delay line is a chain, each link of which is an SRL16 followed by a flip-flop. If register retiming is enabled, the delay line is a chain of flip-flops.  xl_area=[1 1 0 0 0 0 0]  block_version=VER_STRING_GOES_HERE
  Inverter : xbsIndex_r4/Inverter  en=off  latency=0  dbl_ovrd=off  block_type=inv
      settings: xl_area=[0 0 0 0 0 0 0]  block_version=VER_STRING_GOES_HERE
  Logical : xbsIndex_r4/Logical  logical_function=AND  inputs=2  en=off  latency=0  precision=Full  arith_type=Unsigned  n_bits=8  bin_pt=2  align_bp=on  dbl_ovrd=off  block_type=logical
      settings: xl_area=[1 0 0 1 0 0 0]  block_version=VER_STRING_GOES_HERE
  Q : Outport
  wires:
  Logical/1 -> Q/1
  D/1 -> Delay/1, Logical/1
  Inverter/1 -> Logical/2
  Delay/1 -> Inverter/1

== system ofdm_txrx_mimo_2x/OFDM Tx MIMO/TxControl/Posedge2 ==
  D : Inport
  Delay : xbsIndex_r4/Delay  en=off  latency=1  dbl_ovrd=off  reg_retiming=off  block_type=delay
      settings: infoedit=Hardware notes: A delay line is a chain, each link of which is an SRL16 followed by a flip-flop. If register retiming is enabled, the delay line is a chain of flip-flops.  xl_area=[1 1 0 0 0 0 0]  block_version=VER_STRING_GOES_HERE
  Inverter : xbsIndex_r4/Inverter  en=off  latency=0  dbl_ovrd=off  block_type=inv
      settings: xl_area=[0 0 0 0 0 0 0]  block_version=VER_STRING_GOES_HERE
  Logical : xbsIndex_r4/Logical  logical_function=AND  inputs=2  en=off  latency=0  precision=Full  arith_type=Unsigned  n_bits=1  bin_pt=0  align_bp=on  dbl_ovrd=off  block_type=logical
      settings: xl_area=[1 0 0 1 0 0 0]  block_version=VER_STRING_GOES_HERE
  Q : Outport
  wires:
  Delay/1 -> Inverter/1
  Inverter/1 -> Logical/2
  D/1 -> Logical/1, Delay/1
  Logical/1 -> Q/1

== system ofdm_txrx_mimo_2x/OFDM Tx MIMO/TxControl/Start/Done Control ==
  TxDone : Inport
  Start : Inport  Port=2
  Constant2 : xbsIndex_r4/Constant  arith_type=Boolean  const=1  n_bits=1  bin_pt=0  explicit_period=off  period=1  equ=P=C  opselect=C  inp2=PCIN>>17  opr=+  inp1=P  carry=CIN  dbl_ovrd=off  block_type=constant
      settings: block_version=VER_STRING_GOES_HERE
  Convert1 : xbsIndex_r4/Convert  arith_type=Boolean  n_bits=16  bin_pt=14  quantization=Truncate  overflow=Wrap  latency=0  dbl_ovrd=off  pipeline=off  block_type=convert
      settings: block_version=8.2.02
  Convert2 : xbsIndex_r4/Convert  arith_type=Boolean  n_bits=16  bin_pt=14  quantization=Truncate  overflow=Wrap  latency=0  dbl_ovrd=off  pipeline=off  block_type=convert
      settings: block_version=8.2.02
  Inverter : xbsIndex_r4/Inverter  en=off  latency=0  dbl_ovrd=off  block_type=inv
      settings: block_version=8.2.02
  Logical1 : xbsIndex_r4/Logical  logical_function=OR  inputs=2  en=off  latency=0  precision=Full  arith_type=Unsigned  n_bits=8  bin_pt=2  align_bp=on  dbl_ovrd=off  block_type=logical
      settings: xl_area=[1 0 0 1 0 0 0]  block_version=VER_STRING_GOES_HERE
  Logical4 : xbsIndex_r4/Logical  logical_function=AND  inputs=2  en=off  latency=0  precision=Full  arith_type=Unsigned  n_bits=8  bin_pt=2  align_bp=on  dbl_ovrd=off  block_type=logical
      settings: xl_area=[1 0 0 1 0 0 0]  block_version=VER_STRING_GOES_HERE
  Posedge2 : Subsystem
  Posedge3 : Subsystem
  Reg : xbsIndex_r4/Register  init=0  rst=off  en=off  dbl_ovrd=off  block_type=register
      settings: xl_area=[1 1 0 0 0 0 0]  block_version=VER_STRING_GOES_HERE
  S-R Latch : Subsystem
  Tx_PktDone : xbsIndex_r4/Gateway Out  hdl_port=on  timing_constraint=None  locs_specified=off  LOCs={}  block_type=gatewayout
      settings: infoedit="Gateway out block.  Converts Xilinx fixed point inputs into ouputs of type Simulink integer, double, or fixed point.<P><P>Hardware notes:  In hardware these blocks become top level output ports or are discarded, depending on how they are configured."  xl_area=[0 0 0 0 1 0 0]  block_version=VER_STRING_GOES_HERE
  Up Sample1 : xbsIndex_r4/Up Sample  sample_ratio=2  copy_samples=on  dbl_ovrd=off  block_type=usamp
      settings: infoedit="Up samples input data.  Inserted values  can be zeros or copies of the most recent input sample.<P><P>Hardware notes: No hardware is needed if inserted values are copies of the input sample; otherwise, a mux and single bit flip-flop are used."  block_version=VER_STRING_GOES_HERE
  Up Sample2 : xbsIndex_r4/Up Sample  sample_ratio=2  copy_samples=on  dbl_ovrd=off  block_type=usamp
      settings: infoedit="Up samples input data.  Inserted values  can be zeros or copies of the most recent input sample.<P><P>Hardware notes: No hardware is needed if inserted values are copies of the input sample; otherwise, a mux and single bit flip-flop are used."  block_version=VER_STRING_GOES_HERE
  TxStart : Outport
  tag scope: regTx_PktRunning=global, regTx_PktRunningEn=global
  wires:
  Start/1 -> Posedge2/1(D)
  TxDone/1 -> Posedge3/1(D)
  Logical1/1 -> S-R Latch/2(R)
  [TxReset] -> Logical1/2
  S-R Latch/1(Q) -> Up Sample1/1, Inverter/1
  Constant2/1 -> [regTx_PktRunningEn]
  Up Sample1/1 -> [regTx_PktRunning]
  Inverter/1 -> Convert2/1
  Logical4/1 -> TxStart/1
  Posedge3/1(Q) -> Logical1/1, Up Sample2/1
  Convert1/1 -> Logical4/1
  Convert2/1 -> Logical4/2
  Up Sample2/1 -> Reg/1
  Posedge2/1(Q) -> S-R Latch/1(S), Convert1/1
  Reg/1 -> Tx_PktDone/1

== system ofdm_txrx_mimo_2x/OFDM Tx MIMO/TxStart/Copy_Downsample ==
  D : Inport
  Delay : xbsIndex_r4/Delay  en=off  latency=1  dbl_ovrd=off  reg_retiming=off  block_type=delay
      settings: infoedit=Hardware notes: A delay line is a chain, each link of which is an SRL16 followed by a flip-flop. If register retiming is enabled, the delay line is a chain of flip-flops.  block_version=VER_STRING_GOES_HERE
  Down Sample : xbsIndex_r4/Down Sample  sample_ratio=2  sample_phase="Last Value of Frame  (most efficient)"  en=off  latency=1  dbl_ovrd=off  block_type=dsamp
      settings: block_version=VER_STRING_GOES_HERE
  Logical : xbsIndex_r4/Logical  logical_function=OR  inputs=2  en=off  latency=0  precision=Full  arith_type=Unsigned  n_bits=16  bin_pt=0  align_bp=on  dbl_ovrd=off  block_type=logical
      settings: block_version=VER_STRING_GOES_HERE
  Q : Outport
  wires:
  Down Sample/1 -> Q/1
  Logical/1 -> Down Sample/1
  D/1 -> Logical/2, Delay/1
  Delay/1 -> Logical/1

== system ofdm_txrx_mimo_2x/Memory-mapped Registers/TxRx Registers/InterruptControl/Posedge ==
  note: Rising Edge Detection
  D : Inport
  Inverter : xbsIndex_r4/Inverter  en=off  latency=1  dbl_ovrd=off  block_type=inv
      settings: xl_area=[0 0 0 0 0 0 0]  block_version=VER_STRING_GOES_HERE
  Logical1 : xbsIndex_r4/Logical  logical_function=AND  inputs=2  en=off  latency=0  precision=Full  arith_type=Unsigned  n_bits=1  bin_pt=0  align_bp=on  dbl_ovrd=off  block_type=logical
      settings: xl_area=[1 0 0 1 0 0 0]  block_version=VER_STRING_GOES_HERE
  Q : Outport
  wires:
  Inverter/1 -> Logical1/1
  Logical1/1 -> Q/1
  D/1 -> Logical1/2, Inverter/1

== system ofdm_txrx_mimo_2x/Memory-mapped Registers/TxRx Registers/InterruptControl/Posedge1 ==
  note: Rising Edge Detection
  D : Inport
  Inverter : xbsIndex_r4/Inverter  en=off  latency=1  dbl_ovrd=off  block_type=inv
      settings: xl_area=[0 0 0 0 0 0 0]  block_version=VER_STRING_GOES_HERE
  Logical1 : xbsIndex_r4/Logical  logical_function=AND  inputs=2  en=off  latency=0  precision=Full  arith_type=Unsigned  n_bits=1  bin_pt=0  align_bp=on  dbl_ovrd=off  block_type=logical
      settings: xl_area=[1 0 0 1 0 0 0]  block_version=VER_STRING_GOES_HERE
  Q : Outport
  wires:
  D/1 -> Inverter/1, Logical1/2
  Logical1/1 -> Q/1
  Inverter/1 -> Logical1/1

== system ofdm_txrx_mimo_2x/Memory-mapped Registers/TxRx Registers/InterruptControl/Posedge2 ==
  note: Rising Edge Detection
  D : Inport
  Inverter : xbsIndex_r4/Inverter  en=off  latency=1  dbl_ovrd=off  block_type=inv
      settings: xl_area=[0 0 0 0 0 0 0]  block_version=VER_STRING_GOES_HERE
  Logical1 : xbsIndex_r4/Logical  logical_function=AND  inputs=2  en=off  latency=0  precision=Full  arith_type=Unsigned  n_bits=1  bin_pt=0  align_bp=on  dbl_ovrd=off  block_type=logical
      settings: xl_area=[1 0 0 1 0 0 0]  block_version=VER_STRING_GOES_HERE
  Q : Outport
  wires:
  Inverter/1 -> Logical1/1
  Logical1/1 -> Q/1
  D/1 -> Logical1/2, Inverter/1

== system ofdm_txrx_mimo_2x/OFDM Rx MIMO/ADC Inputs & Antenna Selection/Antenna Selection/Ant Sel Logic ==
  note: Two reasons to select antenna B:
      | 1) In SISO mode, switching diversity is enabled and antenna B's gains are lower (i.e. its SNR is higher)
      | 2) In SISO mode, switchinv diversity is disabled and user asserts noSwitch_forceAntB config bit
  G(B) < G(A) : Inport
  B_Vin : Inport  Port=2
  Down Sample1 : xbsIndex_r4/Down Sample  sample_ratio=2  sample_phase="Last Value of Frame  (most efficient)"  en=off  latency=1  dbl_ovrd=off  block_type=dsamp
      settings: block_version=8.2.02
  Inverter : xbsIndex_r4/Inverter  en=off  latency=0  dbl_ovrd=off  block_type=inv
      settings: block_version=8.2.02
  Logical1 : xbsIndex_r4/Logical  logical_function=AND  inputs=4  en=off  latency=0  precision=Full  arith_type=Unsigned  n_bits=8  bin_pt=2  align_bp=on  dbl_ovrd=off  block_type=logical
      settings: xl_area=[1 0 0 1 0 0 0]  block_version=VER_STRING_GOES_HERE
  Logical2 : xbsIndex_r4/Logical  logical_function=AND  inputs=3  en=off  latency=0  precision=Full  arith_type=Unsigned  n_bits=8  bin_pt=2  align_bp=on  dbl_ovrd=off  block_type=logical
      settings: xl_area=[1 0 0 1 0 0 0]  block_version=VER_STRING_GOES_HERE
  Logical3 : xbsIndex_r4/Logical  logical_function=OR  inputs=2  en=off  latency=0  precision=Full  arith_type=Unsigned  n_bits=8  bin_pt=2  align_bp=on  dbl_ovrd=off  block_type=logical
      settings: xl_area=[1 0 0 1 0 0 0]  block_version=VER_STRING_GOES_HERE
  AntSel : Outport
  wires:
  [regRx_SISOmode] -> Down Sample1/1
  Logical1/1 -> Logical3/2
  B_Vin/1 -> Logical1/4
  G(B) < G(A)/1 -> Logical1/3
  Down Sample1/1 -> Logical1/1, Logical2/2
  [regRx_switchingDivEn] -> Logical1/2, Inverter/1
  Logical3/1 -> AntSel/1
  [regRx_noSwitch_forceAntB] -> Logical2/1
  Inverter/1 -> Logical2/3
  Logical2/1 -> Logical3/1
